simulink model "MCVT_Integration"  (R2020a)
settings: Solver=ode8  StartTime=0.0  StopTime=T_end  FixedStep=dt  RelTol=1e-3  EnableMultiTasking=on
values from neighbouring files (symbols this model uses):
  assumed_power_voltage = 1.25e2    (= 125)    [MCVT_Input_File.m]    (also in MCVT_Run_QuickCheck.m)
  battery_power_toggle = 1    [MCVT_Input_File.m]    (also in MCVT_Run_QuickCheck.m)
  battery_trickle_charge_param = 0.1    [MCVT_Input_File.m]    (also in MCVT_Run_QuickCheck.m)
  battery_trickle_leakage_param = 0.01    [MCVT_Input_File.m]    (also in MCVT_Run_QuickCheck.m)
  damage_case_id = 3    [MCVT_Input_File.m]    (also in MCVT_Run_QuickCheck.m = 1)
  dt = 1/fs    (= 0.001)    [MCVT_Run_QuickCheck.m]    (also in MCVT_Sim_Set.m)
  dt_2 = 1/fs_2    (= 0.001)    [MCVT_Run_QuickCheck.m]    (also in MCVT_Sim_Set.m)
  dt_3 = 1/fs_3    (= 0.001)    [MCVT_Run_QuickCheck.m]    (also in MCVT_Sim_Set.m)
  dt_5 = 1/fs_5    (= 0.005)    [MCVT_Run_QuickCheck.m]    (also in MCVT_Sim_Set.m)
  dt_8 = 1/fs_8    (= 0.05)    [MCVT_Run_QuickCheck.m]    (also in MCVT_Sim_Set.m)
  dt_9 = 1/fs_9    (= 0.001)    [MCVT_Run_QuickCheck.m]    (also in MCVT_Sim_Set.m)
  dust_met_duration = 3    [MCVT_Input_File.m]    (also in MCVT_Run_QuickCheck.m = 2)
  ECLSS_indoor_set_temperature = 298.15    [MCVT_Input_File.m]    (also in MCVT_Run_QuickCheck.m)
  energy_store_max_o = 150    [MCVT_Input_File.m]    (also in MCVT_Run_QuickCheck.m)
  energy_store_o = 120    [MCVT_Input_File.m]    (also in MCVT_Run_QuickCheck.m)
  fs = 1000    [MCVT_Run_QuickCheck.m]    (also in MCVT_Sim_Set.m)
  gain_charge_param = 1    [MCVT_Input_File.m]    (also in MCVT_Run_QuickCheck.m)
  ground_temperature = 373    [MCVT_Habitat_Design_Input_File.m]    (also in MCVT_Run_QuickCheck.m)
  I_max_charge = 800    [MCVT_Input_File.m]    (also in MCVT_Run_QuickCheck.m)
  I_max_discharge = 800    [MCVT_Input_File.m]    (also in MCVT_Run_QuickCheck.m)
  initial_pressure = 1e5    (= 100000)    [MCVT_Input_File.m]    (also in MCVT_Run_QuickCheck.m)
  initial_temperature = 295    [MCVT_Input_File.m]    (also in MCVT_Run_QuickCheck.m)
  interior_leak_rate = 0.00415    [MCVT_Input_File.m]    (also in MCVT_Run_QuickCheck.m)
  launch_dust_accum_start_time = 10    [MCVT_Input_File.m]    (also in MCVT_Run_QuickCheck.m = 2)
  launch_dust_accum_stop_time = 12    [MCVT_Input_File.m]    (also in MCVT_Run_QuickCheck.m = 3)
  meteorite_impact_time = 2    [MCVT_Input_File.m]    (also in MCVT_Run_QuickCheck.m = 1)
  nuclear_power_toggle = 1    [MCVT_Input_File.m]    (also in MCVT_Run_QuickCheck.m)
  print_interval = 30    [MCVT_Input_File.m]
  SOC_lower_thres = 0.5    [MCVT_Input_File.m]    (also in MCVT_Run_QuickCheck.m)
  SOC_upper_thres = 0.9    [MCVT_Input_File.m]    (also in MCVT_Run_QuickCheck.m)
  solar_power_toggle = 1    [MCVT_Input_File.m]    (also in MCVT_Run_QuickCheck.m)
  space_temperature = 4    [MCVT_Habitat_Design_Input_File.m]    (also in MCVT_Run_QuickCheck.m)
  Start_angle = 90    [MCVT_Input_File.m]    (also in MCVT_Run_QuickCheck.m)
undefined symbols (not defined in the file or in its folder): A_flow_restrictor, ACTIVITY_LOOKUP_TABLE, air, Aref, Bmap, Bmap2, Bmap3, comms, DiscIntNeverNeededParam, DmgSPL, dust_parameters, ECLSS_dust_damage_repair_time, ECLSS_heater_capacity, ECLSS_IPS_restrictor, ECLSS_IPS_setpointPressure, ECLSS_paint_damage_repair_time, ECLSS_radiatorLoop_discreteNumber, ECLSS_Vst, emptyActivity, expected_heat_add, FI, habitat, HIEM2SMM, HIEM2STM, HIEMDamageElements, impact_numbering, initial_SPLT_damage_index, input_air, input_Cond, input_CP, input_discretization, input_EV, input_Evap, input_radiatorLoop, K_ECLSS_dust_single, K_ECLSS_paint_single, K_Structure_damage_single, Kref, kW_to_W, meteoroidDustDep, mFRF, ndelays, nominalDustDep, noverlap, nuclear_toggle_gain, output_limits, panel_paint0, Ps0, reference_temperature, RepairTime_ECLSS_factor, Sensor_Location_SMM, SMM_T0, SMM_T1, SMM_T2, SMM_T3, SMM_T4, SMM_T4_0, SMM_T4_1, SMM_T4_2, solar_FDD_K ... (+73)
libraries: neural x350, simulink x39, xpcutilitieslib x7, xpcrtudplib x7, dspstat3 x1
(part 10 of 43)

== system MCVT_Integration/Sys2 - Structural System/Sys2_Structural_System/Fault Detection and Diagnosis (FDD) with Binary Classifer/Pattern Recognition Neural Network/Segment 1/Process Output 1 ==
  a : Inport  PortDimensions=2
      mask Display: e = 0.06;c1 = [120 230 180]/255;c2 = ([120 230 180]+0)/(256+128);c3 = ([120 230 180]+128)/(256+128);patch([0 1 1 0],[0 0 1 1],c1);patch([0 1 1 1-e 1-e 0+e],[0 0 1 1-e 0+e 0+e],c2);patch([0 0 1 1-e 0+e 0+e],[0 1 1 1-e 1-e 0+e],c3);plot([0 1 1 0 0],[0 0 1 1 0]);
  y : Outport  PortDimensions=2
      mask Display: e = 0.06;c1 = [120 230 180]/255;c2 = ([120 230 180]+0)/(256+128);c3 = ([120 230 180]+128)/(256+128);patch([0 1 1 0],[0 0 1 1],c1);patch([0 1 1 1-e 1-e 0+e],[0 0 1 1-e 0+e 0+e],c2);patch([0 0 1 1-e 0+e 0+e],[0 1 1 1-e 1-e 0+e],c3);plot([0 1 1 0 0],[0 0 1 1 0]);
  wires:
  a/1 -> y/1

== system MCVT_Integration/Sys2 - Structural System/Sys2_Structural_System/Fault Detection and Diagnosis (FDD) with Binary Classifer/Pattern Recognition Neural Network/Segment 2 (2)/Layer 1 ==
  "p{1}" : Inport  PortDimensions=2304
      mask Display: e = 0.06;c1 = [120 230 180]/255;c2 = ([120 230 180]+0)/(256+128);c3 = ([120 230 180]+128)/(256+128);patch([0 1 1 0],[0 0 1 1],c1);patch([0 1 1 1-e 1-e 0+e],[0 0 1 1-e 0+e 0+e],c2);patch([0 0 1 1-e 0+e 0+e],[0 1 1 1-e 1-e 0+e],c3);plot([0 1 1 0 0],[0 0 1 1 0]);
  Delays 1 : Subsystem
      mask Display: e = 0.06;c1 = [120 150 230]/255;c2 = ([120 150 230]+0)/383;c3 = ([120 150 230]+128)/383;patch([0 1 1 0],[0 0 1 1],c1);patch([0 1 1 1-e 1-e 0+e],[0 0 1 1-e 0+e 0+e],c2);patch([0 0 1 1-e 0+e 0+e],[0 1 1 1-e 1-e 0+e],c3);plot([0 1 1 0 0],[0 0 1 1 0]);disp('0');
  "IW{1,1}" : Subsystem
      mask Display: e = 0.06;c1 = [120 150 230]/255;c2 = ([120 150 230]+0)/383;c3 = ([120 150 230]+128)/383;patch([0 1 1 0],[0 0 1 1],c1);patch([0 1 1 1-e 1-e 0+e],[0 0 1 1-e 0+e 0+e],c2);patch([0 0 1 1-e 0+e 0+e],[0 1 1 1-e 1-e 0+e],c3);plot([0 1 1 0 0],[0 0 1 1 0]);disp('W');
  "b{1}" : Constant  Value=[-1.4076515344643965033100130312959663569927215576171875;1.2507294903452030876422895744326524436473846435546875;1.1014165334614840485727427221718244254589080810546875;-0.9535005338732520652200719268876127898693084716796875;0.80485037228833189448806706423056311905384063720703125;-0.66860572654444616613744756250525824725627899169921875;-0.5144833333955813881033236611983738839626312255859375;-0.382214610286095268776307420921511948108673095703125;-0.2210296978680166557484909617414814420044422149658203125;0.07689014760309638429891521127501619048416614532470703125;0.077336931105068840164307175655267201364040374755859375;0.2189846359883272086666039513147552497684955596923828125;0.362804728015978128485841125439037568867206573486328125;-0.51389763449111625082110776929766871035099029541015625;-0.6560464416534708131933939512236975133419036865234375;0.802942446657271435839220430352725088596343994140625;0.9598192718400699874337078654207289218902587890625;-1.1046573317000838443391330656595528125762939453125;1.2410412508102541817578412519651465117931365966796875;-1.4001049930291367129342461339547298848628997802734375]
      mask Display: e = 0.06;c1 = [120 150 230]/255;c2 = ([120 150 230]+0)/383;c3 = ([120 150 230]+128)/383;patch([0 1 1 0],[0 0 1 1],c1);patch([0 1 1 1-e 1-e 0+e],[0 0 1 1-e 0+e 0+e],c2);patch([0 0 1 1-e 0+e 0+e],[0 1 1 1-e 1-e 0+e],c3);plot([0 1 1 0 0],[0 0 1 1 0]);disp('b');
  netsum : Sum  Inputs=2  InputSameDT=on  SaturateOnIntegerOverflow=on  OutDataTypeStr=Inherit: Same as first input
      mask Display: e = 0.06;c1 = [120 150 230]/255;c2 = ([120 150 230]+0)/383;c3 = ([120 150 230]+128)/383;patch([0 1 1 0],[0 0 1 1],c1);patch([0 1 1 1-e 1-e 0+e],[0 0 1 1-e 0+e 0+e],c2);patch([0 0 1 1-e 0+e 0+e],[0 1 1 1-e 1-e 0+e],c3);plot([0 1 1 0 0],[0 0 1 1 0]);disp('+');
  tansig : neural/Transfer Functions/tansig
  "a{1}" : Outport  InitialOutput=[0;0;0;0;0;0;0;0;0;0;0;0;0;0;0;0;0;0;0;0]
      mask Display: e = 0.06;c1 = [120 230 180]/255;c2 = ([120 230 180]+0)/(256+128);c3 = ([120 230 180]+128)/(256+128);patch([0 1 1 0],[0 0 1 1],c1);patch([0 1 1 1-e 1-e 0+e],[0 0 1 1-e 0+e 0+e],c2);patch([0 0 1 1-e 0+e 0+e],[0 1 1 1-e 1-e 0+e],c3);plot([0 1 1 0 0],[0 0 1 1 0]);
  wires:
  "p{1}"/1 -> Delays 1/1("p{1}")
  Delays 1/1("pd{1,1}") -> "IW{1,1}"/1("pd{1,1}")
  "IW{1,1}"/1("iz{1,1}") -> netsum/1
  "b{1}"/1 -> netsum/2
  netsum/1 -> tansig/1
  tansig/1 -> "a{1}"/1

== system MCVT_Integration/Sys2 - Structural System/Sys2_Structural_System/Fault Detection and Diagnosis (FDD) with Binary Classifer/Pattern Recognition Neural Network/Segment 2 (2)/Layer 2 ==
  "a{1} " : Inport  PortDimensions=20
      mask Display: e = 0.06;c1 = [120 230 180]/255;c2 = ([120 230 180]+0)/(256+128);c3 = ([120 230 180]+128)/(256+128);patch([0 1 1 0],[0 0 1 1],c1);patch([0 1 1 1-e 1-e 0+e],[0 0 1 1-e 0+e 0+e],c2);patch([0 0 1 1-e 0+e 0+e],[0 1 1 1-e 1-e 0+e],c3);plot([0 1 1 0 0],[0 0 1 1 0]);
  Delays 1 : Subsystem
      mask Display: e = 0.06;c1 = [120 150 230]/255;c2 = ([120 150 230]+0)/383;c3 = ([120 150 230]+128)/383;patch([0 1 1 0],[0 0 1 1],c1);patch([0 1 1 1-e 1-e 0+e],[0 0 1 1-e 0+e 0+e],c2);patch([0 0 1 1-e 0+e 0+e],[0 1 1 1-e 1-e 0+e],c3);plot([0 1 1 0 0],[0 0 1 1 0]);disp('0');
  "LW{2,1}" : Subsystem
      mask Display: e = 0.06;c1 = [120 150 230]/255;c2 = ([120 150 230]+0)/383;c3 = ([120 150 230]+128)/383;patch([0 1 1 0],[0 0 1 1],c1);patch([0 1 1 1-e 1-e 0+e],[0 0 1 1-e 0+e 0+e],c2);patch([0 0 1 1-e 0+e 0+e],[0 1 1 1-e 1-e 0+e],c3);plot([0 1 1 0 0],[0 0 1 1 0]);disp('W');
  "b{2}" : Constant  Value=[1.616259074734227141334486077539622783660888671875;-1.4491846677885094063498172545223496854305267333984375;-1.2848485960108513292965426444425247609615325927734375;1.1157131012748624510066974835353903472423553466796875;0.9437806136996143013817572864354588091373443603515625;0.76973255481434843527921429995330981910228729248046875;0.6017408575884986010606780837406404316425323486328125;-0.42380146554234432443308833171613514423370361328125;-0.256865652769475205641214188290177844464778900146484375;-0.0916742495315256700205708284556749276816844940185546875;-0.0938883942322570330674125216319225728511810302734375;-0.257489027951350257783502684105769731104373931884765625;-0.427852246789971690787979241576977074146270751953125;0.589954907078594548153205323615111410617828369140625;0.7864551765408631656129045950365252792835235595703125;0.933533742804836919049193966202437877655029296875;1.1090057916075510835440809387364424765110015869140625;-1.2764809705882733670279094440047629177570343017578125;-1.448903619900048678204029783955775201320648193359375;1.6209909591608908652204945610719732940196990966796875]
      mask Display: e = 0.06;c1 = [120 150 230]/255;c2 = ([120 150 230]+0)/383;c3 = ([120 150 230]+128)/383;patch([0 1 1 0],[0 0 1 1],c1);patch([0 1 1 1-e 1-e 0+e],[0 0 1 1-e 0+e 0+e],c2);patch([0 0 1 1-e 0+e 0+e],[0 1 1 1-e 1-e 0+e],c3);plot([0 1 1 0 0],[0 0 1 1 0]);disp('b');
  netsum : Sum  Inputs=2  InputSameDT=on  SaturateOnIntegerOverflow=on  OutDataTypeStr=Inherit: Same as first input
      mask Display: e = 0.06;c1 = [120 150 230]/255;c2 = ([120 150 230]+0)/383;c3 = ([120 150 230]+128)/383;patch([0 1 1 0],[0 0 1 1],c1);patch([0 1 1 1-e 1-e 0+e],[0 0 1 1-e 0+e 0+e],c2);patch([0 0 1 1-e 0+e 0+e],[0 1 1 1-e 1-e 0+e],c3);plot([0 1 1 0 0],[0 0 1 1 0]);disp('+');
  tansig : neural/Transfer Functions/tansig
  "a{2}" : Outport  InitialOutput=[0;0;0;0;0;0;0;0;0;0;0;0;0;0;0;0;0;0;0;0]
      mask Display: e = 0.06;c1 = [120 230 180]/255;c2 = ([120 230 180]+0)/(256+128);c3 = ([120 230 180]+128)/(256+128);patch([0 1 1 0],[0 0 1 1],c1);patch([0 1 1 1-e 1-e 0+e],[0 0 1 1-e 0+e 0+e],c2);patch([0 0 1 1-e 0+e 0+e],[0 1 1 1-e 1-e 0+e],c3);plot([0 1 1 0 0],[0 0 1 1 0]);
  wires:
  "a{1} "/1 -> Delays 1/1("p{2}")
  Delays 1/1("pd{2,1}") -> "LW{2,1}"/1("ad{2,1}")
  "LW{2,1}"/1("lz{2,1}") -> netsum/1
  "b{2}"/1 -> netsum/2
  netsum/1 -> tansig/1
  tansig/1 -> "a{2}"/1

== system MCVT_Integration/Sys2 - Structural System/Sys2_Structural_System/Fault Detection and Diagnosis (FDD) with Binary Classifer/Pattern Recognition Neural Network/Segment 2 (2)/Layer 3 ==
  "a{2} " : Inport  PortDimensions=20
      mask Display: e = 0.06;c1 = [120 230 180]/255;c2 = ([120 230 180]+0)/(256+128);c3 = ([120 230 180]+128)/(256+128);patch([0 1 1 0],[0 0 1 1],c1);patch([0 1 1 1-e 1-e 0+e],[0 0 1 1-e 0+e 0+e],c2);patch([0 0 1 1-e 0+e 0+e],[0 1 1 1-e 1-e 0+e],c3);plot([0 1 1 0 0],[0 0 1 1 0]);
  Delays 1 : Subsystem
      mask Display: e = 0.06;c1 = [120 150 230]/255;c2 = ([120 150 230]+0)/383;c3 = ([120 150 230]+128)/383;patch([0 1 1 0],[0 0 1 1],c1);patch([0 1 1 1-e 1-e 0+e],[0 0 1 1-e 0+e 0+e],c2);patch([0 0 1 1-e 0+e 0+e],[0 1 1 1-e 1-e 0+e],c3);plot([0 1 1 0 0],[0 0 1 1 0]);disp('0');
  "LW{3,2}" : Subsystem
      mask Display: e = 0.06;c1 = [120 150 230]/255;c2 = ([120 150 230]+0)/383;c3 = ([120 150 230]+128)/383;patch([0 1 1 0],[0 0 1 1],c1);patch([0 1 1 1-e 1-e 0+e],[0 0 1 1-e 0+e 0+e],c2);patch([0 0 1 1-e 0+e 0+e],[0 1 1 1-e 1-e 0+e],c3);plot([0 1 1 0 0],[0 0 1 1 0]);disp('W');
  "b{3}" : Constant  Value=[-0.9165786325189682059999540797434747219085693359375;0.84002991411300664825745343478047288954257965087890625]
      mask Display: e = 0.06;c1 = [120 150 230]/255;c2 = ([120 150 230]+0)/383;c3 = ([120 150 230]+128)/383;patch([0 1 1 0],[0 0 1 1],c1);patch([0 1 1 1-e 1-e 0+e],[0 0 1 1-e 0+e 0+e],c2);patch([0 0 1 1-e 0+e 0+e],[0 1 1 1-e 1-e 0+e],c3);plot([0 1 1 0 0],[0 0 1 1 0]);disp('b');
  netsum : Sum  Inputs=2  InputSameDT=on  SaturateOnIntegerOverflow=on  OutDataTypeStr=Inherit: Same as first input
      mask Display: e = 0.06;c1 = [120 150 230]/255;c2 = ([120 150 230]+0)/383;c3 = ([120 150 230]+128)/383;patch([0 1 1 0],[0 0 1 1],c1);patch([0 1 1 1-e 1-e 0+e],[0 0 1 1-e 0+e 0+e],c2);patch([0 0 1 1-e 0+e 0+e],[0 1 1 1-e 1-e 0+e],c3);plot([0 1 1 0 0],[0 0 1 1 0]);disp('+');
  softmax : neural/Transfer Functions/softmax
      settings: SourceProductBaseCode=NN
  "a{3}" : Outport  InitialOutput=[0;0]
      mask Display: e = 0.06;c1 = [120 230 180]/255;c2 = ([120 230 180]+0)/(256+128);c3 = ([120 230 180]+128)/(256+128);patch([0 1 1 0],[0 0 1 1],c1);patch([0 1 1 1-e 1-e 0+e],[0 0 1 1-e 0+e 0+e],c2);patch([0 0 1 1-e 0+e 0+e],[0 1 1 1-e 1-e 0+e],c3);plot([0 1 1 0 0],[0 0 1 1 0]);
  wires:
  "a{2} "/1 -> Delays 1/1("p{3}")
  Delays 1/1("pd{3,2}") -> "LW{3,2}"/1("ad{3,2}")
  "LW{3,2}"/1("lz{3,2}") -> netsum/1
  "b{3}"/1 -> netsum/2
  netsum/1 -> softmax/1
  softmax/1 -> "a{3}"/1
